simulink model "TosAeb"  (R2021a)
settings: Solver=FixedStepDiscrete  StartTime=0.0  StopTime=inf  FixedStep=0.01  RelTol=1e-3
undefined symbols (not defined in the file or in its folder): ACCModSts, AccSts1, AEB_ObjectMovingOrientation, AEB_ObjectMovingState, CN_BTCActive, CN_CurrentGearR, CN_CurrentGearR_DHT, CN_PTCActive, CN_VDCActive, CN_VSC_EspOff, CN_VSC_FCW_AEB_Error, CN_VSC_FCW_AEB_NoDispay, CN_VSC_FCW_AEB_PerfmDgdtn, CN_VSC_FCW_AEB_TempUnAvlbl, CN_VSC_VehStandstill, CNaccelAvoidMax, CNaccelAvoidMin, CNbrakePedalPress, CNcomfortStrLeftAccelMax, CNcomfortStrLeftAccelMin, CNcomfortStrRightAccelMax, CNcomfortStrRightAccelMin, CNDrvDoorOpen, CNDrvModeEco, CNDrvModeNorm, CNDrvModeOther, CNDrvModeSport, CNDrvSBRreminder, CNNull, CNobjInvalidID, CNPassDoorOpen, CNPassSBRreminder, CNPEBSAccelNegInf, CNPEBSAccelNull, CNPEBSTimeInf, CNPEBSTimeNull, CNSTOPMAX, CNSTOPNULL, CNsubjWidth, CNSwtOFF, CNSwtON, CNTTCMAX, CNTTCNULL, CNVehConfigAEBCycNone, CNVehConfigAEBNone, CNVehConfigAEBPedNone, CNVehConfigFCWNone, CNVehConfigJANone, CollisionSeverity, FALSE, FCWSnvty, JACycObjCalcInfoDefaultParams, JAPedObjCalcInfoDefaultParams, JAVehObjCalcInfoDefaultParams, latCycObjCalcInfoDefaultParams, latPedObjCalcInfoDefaultParams, longObjCalcInfoDefaultParams, ModSts, movingStatus, ObjectType ... (+1112)
libraries: simulink x3031, AEBSFunctionLib x50, Ta_Library x33, AEBSStateMachineLib x23, Mylibrary x12, simulink_extras x7, ObjSelect_lib x3, dspstat3 x2
(part 91 of 102)

== system TosAeb/AEB_Objsel/TA_Processor/AEB_ObjSelect/TargetInfoCheck/Data_precess/TA_EffectCalculate/Obj_FilterFlgCalculate/Long_ObjFilter/TTR_Calculate/CalculateTTRAndTTM_else/LongitudinalTTRComputer/CalculateTTI/StationaryHost/CheckIfSolutionExists/LimitedSafeDivide/Saturation Dynamic ==
  up : Inport
  u : Inport  Port=2
  lo : Inport  Port=3
  Data Type Duplicate : DataTypeDuplicate  DisableCoverage=on
  Data Type Propagation : S-Function  FunctionName=sfix_dtprop  Parameters=14,PropDataTypeMode,(getdatatypespecs(PropDataType,PropScaling,DblOver,0, 0, PropDataTypeMode, PropScalingMode)),IfRefDouble,IfRefSingle,IsSigned,NumBitsBase,NumBitsMult,NumBitsAdd,(sort(reshape(round(NumBitsAllowFinal), prod(size(NumBitsAllowFinal)),1))),PropScalingMode,ValuesUsedBestPrec,SlopeBase,SlopeMult,SlopeAdd,BiasBase,BiasMult,BiasAdd,DblOver  SFunctionDeploymentMode=off  EnableBusSupport=off  mask(Data Type Propagation){PropDataTypeMode=Inherit via propagation rule, PropDataType=fixdt(1, 16), IfRefDouble=double, IfRefSingle=single, IsSigned=IsSigned1, NumBitsBase=NumBits1, NumBitsMult=1, NumBitsAdd=0, NumBitsAllowFinal=1:128, PropScalingMode=Inherit via propagation rule, PropScaling=2^-10, ValuesUsedBestPrec=[5 -7], SlopeBase=Slope1, SlopeMult=1, SlopeAdd=0, BiasBase=Bias1, BiasMult=1, BiasAdd=0}
      mask prompts: PropDataTypeMode "Simulink:masks:x1PropagatedDataType_MP"; PropDataType "Simulink:masks:x11PropagatedDataTypeegFixdt116Fixdtsingle_MP"; IfRefDouble "Simulink:masks:x11IfAnyReferenceInputIsDoubleOutputIs_MP"; IfRefSingle "Simulink:masks:x12IfAnyReferenceInputIsSingleOutputIs_MP"; IsSigned "Simulink:masks:x13IsSigned_MP"; NumBitsBase "Simulink:masks:x141NumberofBitsBase_MP"; NumBitsMult "Simulink:masks:x142NumberofBitsMultiplicativeAdjustment_MP"; NumBitsAdd "Simulink:masks:x143NumberofBitsAdditiveAdjustment_MP"; NumBitsAllowFinal "Simulink:masks:x144NumberofBitsAllowableFinalValues_MP"; PropScalingMode "Simulink:masks:x2PropagatedScaling_MP"; PropScaling "Simulink:masks:x21PropagatedScalingSlopeEg29OrSlopeBiasEg1253_MP"; ValuesUsedBestPrec "Simulink:masks:x21ValuesUsedToDetermineBestPrecisionScaling_MP"; SlopeBase "Simulink:masks:x211SlopeBase_MP"; SlopeMult "Simulink:masks:x212SlopeMultiplicativeAdjustment_MP"; SlopeAdd "Simulink:masks:x213SlopeAdditiveAdjustment_MP"; BiasBase "Simulink:masks:x221BiasBase_MP"; BiasMult "Simulink:masks:x222BiasMultiplicativeAdjustment_MP"; BiasAdd "Simulink:masks:x223BiasAdditiveAdjustment_MP"
      mask help: Simulink:masks:DataTypePropagation_MD
      mask init:
      | DblOver = 0;
      mask TypeOptions PropDataTypeMode: Simulink:masks:SpecifyViaDialog | Simulink:masks:InheritViaPropagationRule
      mask Callback PropDataTypeMode: fixptdialog(gcbh)
      mask Callback PropDataType: fixptdialog(gcbh)
      mask TypeOptions IfRefDouble: Simulink:masks:double | Simulink:masks:single
      mask TypeOptions IfRefSingle: Simulink:masks:double | Simulink:masks:single
      mask TypeOptions IsSigned: Simulink:masks:IsSigned1 | Simulink:masks:IsSigned2 | Simulink:masks:IsSigned1OrIsSigned2 | Simulink:masks:TRUE | Simulink:masks:FALSE
      mask TypeOptions NumBitsBase: NumBits1 | NumBits2 | max([NumBits1 NumBits2]) | min([NumBits1 NumBits2]) | NumBits1+NumBits2
      mask TypeOptions PropScalingMode: Simulink:masks:SpecifyViaDialog | Simulink:masks:InheritViaPropagationRule | Simulink:masks:ObtainViaBestPrecision
      mask Callback PropScalingMode: fixptdialog(gcbh)
      mask TypeOptions SlopeBase: Slope1 | Slope2 | max([Slope1 Slope2]) | min([Slope1 Slope2]) | Slope1*Slope2 | Slope1/Slope2 | PosRange1 | PosRange2 | max([PosRange1 PosRange2]) | min([PosRange1 PosRange2]) | PosRange1*PosRange2 | PosRange1/PosRange2
      mask TypeOptions BiasBase: Bias1 | Bias2 | max([Bias1 Bias2]) | min([Bias1 Bias2]) | Bias1*Bias2 | Bias1/Bias2 | Bias1+Bias2 | Bias1-Bias2
      mask Display:
      | port_label('Input',1,'Ref1');
      | port_label('Input',2,'Ref2');
      | port_label('Input',3,'Prop');
  LowerRelop1 : RelationalOperator  DisableCoverage=on  Operator=>  OutDataTypeStr=boolean  RndMeth=Nearest
  Switch : Switch  Criteria=u2 ~= 0  OutDataTypeStr=Inherit: Inherit via back propagation  ZeroCross=off
  Switch2 : Switch  Criteria=u2 ~= 0  OutMin=OutMin  OutMax=OutMax  OutDataTypeStr=Inherit: Inherit via back propagation  ZeroCross=off
  UpperRelop : RelationalOperator  DisableCoverage=on  Operator=<  OutDataTypeStr=boolean  RndMeth=Nearest
  y : Outport  OutMin=OutMin  OutMax=OutMax
  wires:
  up/1 -> Switch2/1, LowerRelop1/2
  u/1 -> Data Type Propagation/2, Data Type Propagation/1, LowerRelop1/1, Switch/3, UpperRelop/1
  lo/1 -> Switch/1, UpperRelop/2
  Switch/1 -> Data Type Duplicate/2, Switch2/3, Data Type Propagation/3
  Switch2/1 -> Data Type Duplicate/1, y/1
  LowerRelop1/1 -> Switch2/2
  UpperRelop/1 -> Switch/2

== system TosAeb/AEB_Objsel/TA_Processor/AEB_ObjSelect/TargetInfoCheck/Data_precess/TA_EffectCalculate/Obj_FilterFlgCalculate/Long_ObjFilter/TTR_Calculate/CalculateTTRAndTTM_else/LongitudinalTTRComputer/CalculateTTI/StationaryHost/CheckIfSolutionExists1/LimitedSafeDivide/SafeDivide ==
  Numerator : Inport  OutDataTypeStr=DataType
      settings: AttributesFormatString=DT=%<OutDataTypeStr>
  Denominator : Inport  Port=2  OutDataTypeStr=DataType
      settings: AttributesFormatString=DT=%<OutDataTypeStr>
  SafeDivide : MATLAB Function
  Quotient : Outport  OutDataTypeStr=DataType
      settings: AttributesFormatString=DT=%<OutDataTypeStr>
  wires:
  SafeDivide/1(Quotient) -> Quotient/1
  Denominator/1 -> SafeDivide/2(Denominator)  "Denominator"
  Numerator/1 -> SafeDivide/1(Numerator)  "Numerator"

== system TosAeb/AEB_Objsel/TA_Processor/AEB_ObjSelect/TargetInfoCheck/Data_precess/TA_EffectCalculate/Obj_FilterFlgCalculate/Long_ObjFilter/TTR_Calculate/CalculateTTRAndTTM_else/LongitudinalTTRComputer/CalculateTTI/StationaryHost/CheckIfSolutionExists1/LimitedSafeDivide/Saturation Dynamic ==
  up : Inport
  u : Inport  Port=2
  lo : Inport  Port=3
  Data Type Duplicate : DataTypeDuplicate  DisableCoverage=on
  Data Type Propagation : S-Function  FunctionName=sfix_dtprop  Parameters=14,PropDataTypeMode,(getdatatypespecs(PropDataType,PropScaling,DblOver,0, 0, PropDataTypeMode, PropScalingMode)),IfRefDouble,IfRefSingle,IsSigned,NumBitsBase,NumBitsMult,NumBitsAdd,(sort(reshape(round(NumBitsAllowFinal), prod(size(NumBitsAllowFinal)),1))),PropScalingMode,ValuesUsedBestPrec,SlopeBase,SlopeMult,SlopeAdd,BiasBase,BiasMult,BiasAdd,DblOver  SFunctionDeploymentMode=off  EnableBusSupport=off  mask(Data Type Propagation){PropDataTypeMode=Inherit via propagation rule, PropDataType=fixdt(1, 16), IfRefDouble=double, IfRefSingle=single, IsSigned=IsSigned1, NumBitsBase=NumBits1, NumBitsMult=1, NumBitsAdd=0, NumBitsAllowFinal=1:128, PropScalingMode=Inherit via propagation rule, PropScaling=2^-10, ValuesUsedBestPrec=[5 -7], SlopeBase=Slope1, SlopeMult=1, SlopeAdd=0, BiasBase=Bias1, BiasMult=1, BiasAdd=0}
      mask prompts: PropDataTypeMode "Simulink:masks:x1PropagatedDataType_MP"; PropDataType "Simulink:masks:x11PropagatedDataTypeegFixdt116Fixdtsingle_MP"; IfRefDouble "Simulink:masks:x11IfAnyReferenceInputIsDoubleOutputIs_MP"; IfRefSingle "Simulink:masks:x12IfAnyReferenceInputIsSingleOutputIs_MP"; IsSigned "Simulink:masks:x13IsSigned_MP"; NumBitsBase "Simulink:masks:x141NumberofBitsBase_MP"; NumBitsMult "Simulink:masks:x142NumberofBitsMultiplicativeAdjustment_MP"; NumBitsAdd "Simulink:masks:x143NumberofBitsAdditiveAdjustment_MP"; NumBitsAllowFinal "Simulink:masks:x144NumberofBitsAllowableFinalValues_MP"; PropScalingMode "Simulink:masks:x2PropagatedScaling_MP"; PropScaling "Simulink:masks:x21PropagatedScalingSlopeEg29OrSlopeBiasEg1253_MP"; ValuesUsedBestPrec "Simulink:masks:x21ValuesUsedToDetermineBestPrecisionScaling_MP"; SlopeBase "Simulink:masks:x211SlopeBase_MP"; SlopeMult "Simulink:masks:x212SlopeMultiplicativeAdjustment_MP"; SlopeAdd "Simulink:masks:x213SlopeAdditiveAdjustment_MP"; BiasBase "Simulink:masks:x221BiasBase_MP"; BiasMult "Simulink:masks:x222BiasMultiplicativeAdjustment_MP"; BiasAdd "Simulink:masks:x223BiasAdditiveAdjustment_MP"
      mask help: Simulink:masks:DataTypePropagation_MD
      mask init:
      | DblOver = 0;
      mask TypeOptions PropDataTypeMode: Simulink:masks:SpecifyViaDialog | Simulink:masks:InheritViaPropagationRule
      mask Callback PropDataTypeMode: fixptdialog(gcbh)
      mask Callback PropDataType: fixptdialog(gcbh)
      mask TypeOptions IfRefDouble: Simulink:masks:double | Simulink:masks:single
      mask TypeOptions IfRefSingle: Simulink:masks:double | Simulink:masks:single
      mask TypeOptions IsSigned: Simulink:masks:IsSigned1 | Simulink:masks:IsSigned2 | Simulink:masks:IsSigned1OrIsSigned2 | Simulink:masks:TRUE | Simulink:masks:FALSE
      mask TypeOptions NumBitsBase: NumBits1 | NumBits2 | max([NumBits1 NumBits2]) | min([NumBits1 NumBits2]) | NumBits1+NumBits2
      mask TypeOptions PropScalingMode: Simulink:masks:SpecifyViaDialog | Simulink:masks:InheritViaPropagationRule | Simulink:masks:ObtainViaBestPrecision
      mask Callback PropScalingMode: fixptdialog(gcbh)
      mask TypeOptions SlopeBase: Slope1 | Slope2 | max([Slope1 Slope2]) | min([Slope1 Slope2]) | Slope1*Slope2 | Slope1/Slope2 | PosRange1 | PosRange2 | max([PosRange1 PosRange2]) | min([PosRange1 PosRange2]) | PosRange1*PosRange2 | PosRange1/PosRange2
      mask TypeOptions BiasBase: Bias1 | Bias2 | max([Bias1 Bias2]) | min([Bias1 Bias2]) | Bias1*Bias2 | Bias1/Bias2 | Bias1+Bias2 | Bias1-Bias2
      mask Display:
      | port_label('Input',1,'Ref1');
      | port_label('Input',2,'Ref2');
      | port_label('Input',3,'Prop');
  LowerRelop1 : RelationalOperator  DisableCoverage=on  Operator=>  OutDataTypeStr=boolean  RndMeth=Nearest
  Switch : Switch  Criteria=u2 ~= 0  OutDataTypeStr=Inherit: Inherit via back propagation  ZeroCross=off
  Switch2 : Switch  Criteria=u2 ~= 0  OutMin=OutMin  OutMax=OutMax  OutDataTypeStr=Inherit: Inherit via back propagation  ZeroCross=off
  UpperRelop : RelationalOperator  DisableCoverage=on  Operator=<  OutDataTypeStr=boolean  RndMeth=Nearest
  y : Outport  OutMin=OutMin  OutMax=OutMax
  wires:
  up/1 -> Switch2/1, LowerRelop1/2
  u/1 -> Data Type Propagation/2, Data Type Propagation/1, LowerRelop1/1, Switch/3, UpperRelop/1
  lo/1 -> Switch/1, UpperRelop/2
  Switch/1 -> Data Type Duplicate/2, Switch2/3, Data Type Propagation/3
  Switch2/1 -> Data Type Duplicate/1, y/1
  LowerRelop1/1 -> Switch2/2
  UpperRelop/1 -> Switch/2

== system TosAeb/AEB_Objsel/TA_Processor/AEB_ObjSelect/TargetInfoCheck/Data_precess/TA_EffectCalculate/Obj_FilterFlgCalculate/Long_ObjFilter/TTR_Calculate/CalculateTTRAndTTM_else/LongitudinalTTRComputer/CalculateTTI/StationaryHost/TimeToIntersectionComputer/SolutionChooser/Compare To Zero1 ==
  u : Inport
  Compare : RelationalOperator  Operator=<  OutDataTypeStr=boolean  RndMeth=Nearest  InputSameDT=on
  Constant : Constant  DisableCoverage=on  Value=0  OutDataTypeStr=Inherit: Inherit via back propagation  PreserveConstantTs=on
  y : Outport
  wires:
  Constant/1 -> Compare/2
  u/1 -> Compare/1
  Compare/1 -> y/1

== system TosAeb/AEB_Objsel/TA_Processor/AEB_ObjSelect/TargetInfoCheck/Data_precess/TA_EffectCalculate/Obj_FilterFlgCalculate/Long_ObjFilter/TTR_Calculate/CalculateTTRAndTTM_else/LongitudinalTTRComputer/CalculateTTI/StationaryHost/TimeToIntersectionComputer1/SolutionChooser/Compare To Zero1 ==
  u : Inport
  Compare : RelationalOperator  Operator=<  OutDataTypeStr=boolean  RndMeth=Nearest  InputSameDT=on
  Constant : Constant  DisableCoverage=on  Value=0  OutDataTypeStr=Inherit: Inherit via back propagation  PreserveConstantTs=on
  y : Outport
  wires:
  Compare/1 -> y/1
  u/1 -> Compare/1
  Constant/1 -> Compare/2

== system TosAeb/AEB_Objsel/TA_Processor/AEB_ObjSelect/TargetInfoCheck/Data_precess/TA_EffectCalculate/Obj_FilterFlgCalculate/Long_ObjFilter/TTR_Calculate/CalculateTTRAndTTM_else/LongitudinalTTRComputer/CalculateTTI/StationaryObject/CheckIfSolutionExists/LimitedSafeDivide/SafeDivide ==
  Numerator : Inport  OutDataTypeStr=DataType
      settings: AttributesFormatString=DT=%<OutDataTypeStr>
  Denominator : Inport  Port=2  OutDataTypeStr=DataType
      settings: AttributesFormatString=DT=%<OutDataTypeStr>
  SafeDivide : MATLAB Function
  Quotient : Outport  OutDataTypeStr=DataType
      settings: AttributesFormatString=DT=%<OutDataTypeStr>
  wires:
  SafeDivide/1(Quotient) -> Quotient/1
  Denominator/1 -> SafeDivide/2(Denominator)  "Denominator"
  Numerator/1 -> SafeDivide/1(Numerator)  "Numerator"

== system TosAeb/AEB_Objsel/TA_Processor/AEB_ObjSelect/TargetInfoCheck/Data_precess/TA_EffectCalculate/Obj_FilterFlgCalculate/Long_ObjFilter/TTR_Calculate/CalculateTTRAndTTM_else/LongitudinalTTRComputer/CalculateTTI/StationaryObject/CheckIfSolutionExists/LimitedSafeDivide/Saturation Dynamic ==
  up : Inport
  u : Inport  Port=2
  lo : Inport  Port=3
  Data Type Duplicate : DataTypeDuplicate  DisableCoverage=on
  Data Type Propagation : S-Function  FunctionName=sfix_dtprop  Parameters=14,PropDataTypeMode,(getdatatypespecs(PropDataType,PropScaling,DblOver,0, 0, PropDataTypeMode, PropScalingMode)),IfRefDouble,IfRefSingle,IsSigned,NumBitsBase,NumBitsMult,NumBitsAdd,(sort(reshape(round(NumBitsAllowFinal), prod(size(NumBitsAllowFinal)),1))),PropScalingMode,ValuesUsedBestPrec,SlopeBase,SlopeMult,SlopeAdd,BiasBase,BiasMult,BiasAdd,DblOver  SFunctionDeploymentMode=off  EnableBusSupport=off  mask(Data Type Propagation){PropDataTypeMode=Inherit via propagation rule, PropDataType=fixdt(1, 16), IfRefDouble=double, IfRefSingle=single, IsSigned=IsSigned1, NumBitsBase=NumBits1, NumBitsMult=1, NumBitsAdd=0, NumBitsAllowFinal=1:128, PropScalingMode=Inherit via propagation rule, PropScaling=2^-10, ValuesUsedBestPrec=[5 -7], SlopeBase=Slope1, SlopeMult=1, SlopeAdd=0, BiasBase=Bias1, BiasMult=1, BiasAdd=0}
      mask prompts: PropDataTypeMode "Simulink:masks:x1PropagatedDataType_MP"; PropDataType "Simulink:masks:x11PropagatedDataTypeegFixdt116Fixdtsingle_MP"; IfRefDouble "Simulink:masks:x11IfAnyReferenceInputIsDoubleOutputIs_MP"; IfRefSingle "Simulink:masks:x12IfAnyReferenceInputIsSingleOutputIs_MP"; IsSigned "Simulink:masks:x13IsSigned_MP"; NumBitsBase "Simulink:masks:x141NumberofBitsBase_MP"; NumBitsMult "Simulink:masks:x142NumberofBitsMultiplicativeAdjustment_MP"; NumBitsAdd "Simulink:masks:x143NumberofBitsAdditiveAdjustment_MP"; NumBitsAllowFinal "Simulink:masks:x144NumberofBitsAllowableFinalValues_MP"; PropScalingMode "Simulink:masks:x2PropagatedScaling_MP"; PropScaling "Simulink:masks:x21PropagatedScalingSlopeEg29OrSlopeBiasEg1253_MP"; ValuesUsedBestPrec "Simulink:masks:x21ValuesUsedToDetermineBestPrecisionScaling_MP"; SlopeBase "Simulink:masks:x211SlopeBase_MP"; SlopeMult "Simulink:masks:x212SlopeMultiplicativeAdjustment_MP"; SlopeAdd "Simulink:masks:x213SlopeAdditiveAdjustment_MP"; BiasBase "Simulink:masks:x221BiasBase_MP"; BiasMult "Simulink:masks:x222BiasMultiplicativeAdjustment_MP"; BiasAdd "Simulink:masks:x223BiasAdditiveAdjustment_MP"
      mask help: Simulink:masks:DataTypePropagation_MD
      mask init:
      | DblOver = 0;
      mask TypeOptions PropDataTypeMode: Simulink:masks:SpecifyViaDialog | Simulink:masks:InheritViaPropagationRule
      mask Callback PropDataTypeMode: fixptdialog(gcbh)
      mask Callback PropDataType: fixptdialog(gcbh)
      mask TypeOptions IfRefDouble: Simulink:masks:double | Simulink:masks:single
      mask TypeOptions IfRefSingle: Simulink:masks:double | Simulink:masks:single
      mask TypeOptions IsSigned: Simulink:masks:IsSigned1 | Simulink:masks:IsSigned2 | Simulink:masks:IsSigned1OrIsSigned2 | Simulink:masks:TRUE | Simulink:masks:FALSE
      mask TypeOptions NumBitsBase: NumBits1 | NumBits2 | max([NumBits1 NumBits2]) | min([NumBits1 NumBits2]) | NumBits1+NumBits2
      mask TypeOptions PropScalingMode: Simulink:masks:SpecifyViaDialog | Simulink:masks:InheritViaPropagationRule | Simulink:masks:ObtainViaBestPrecision
      mask Callback PropScalingMode: fixptdialog(gcbh)
      mask TypeOptions SlopeBase: Slope1 | Slope2 | max([Slope1 Slope2]) | min([Slope1 Slope2]) | Slope1*Slope2 | Slope1/Slope2 | PosRange1 | PosRange2 | max([PosRange1 PosRange2]) | min([PosRange1 PosRange2]) | PosRange1*PosRange2 | PosRange1/PosRange2
      mask TypeOptions BiasBase: Bias1 | Bias2 | max([Bias1 Bias2]) | min([Bias1 Bias2]) | Bias1*Bias2 | Bias1/Bias2 | Bias1+Bias2 | Bias1-Bias2
      mask Display:
      | port_label('Input',1,'Ref1');
      | port_label('Input',2,'Ref2');
      | port_label('Input',3,'Prop');
  LowerRelop1 : RelationalOperator  DisableCoverage=on  Operator=>  OutDataTypeStr=boolean  RndMeth=Nearest
  Switch : Switch  Criteria=u2 ~= 0  OutDataTypeStr=Inherit: Inherit via back propagation  ZeroCross=off
  Switch2 : Switch  Criteria=u2 ~= 0  OutMin=OutMin  OutMax=OutMax  OutDataTypeStr=Inherit: Inherit via back propagation  ZeroCross=off
  UpperRelop : RelationalOperator  DisableCoverage=on  Operator=<  OutDataTypeStr=boolean  RndMeth=Nearest
  y : Outport  OutMin=OutMin  OutMax=OutMax
  wires:
  up/1 -> Switch2/1, LowerRelop1/2
  u/1 -> Data Type Propagation/2, Data Type Propagation/1, LowerRelop1/1, Switch/3, UpperRelop/1
  lo/1 -> Switch/1, UpperRelop/2
  Switch/1 -> Data Type Duplicate/2, Switch2/3, Data Type Propagation/3
  Switch2/1 -> Data Type Duplicate/1, y/1
  LowerRelop1/1 -> Switch2/2
  UpperRelop/1 -> Switch/2

== system TosAeb/AEB_Objsel/TA_Processor/AEB_ObjSelect/TargetInfoCheck/Data_precess/TA_EffectCalculate/Obj_FilterFlgCalculate/Long_ObjFilter/TTR_Calculate/CalculateTTRAndTTM_else/LongitudinalTTRComputer/CalculateTTI/StationaryObject/CheckIfSolutionExists1/LimitedSafeDivide/SafeDivide ==
  Numerator : Inport  OutDataTypeStr=DataType
      settings: AttributesFormatString=DT=%<OutDataTypeStr>
  Denominator : Inport  Port=2  OutDataTypeStr=DataType
      settings: AttributesFormatString=DT=%<OutDataTypeStr>
  SafeDivide : MATLAB Function
  Quotient : Outport  OutDataTypeStr=DataType
      settings: AttributesFormatString=DT=%<OutDataTypeStr>
  wires:
  SafeDivide/1(Quotient) -> Quotient/1
  Denominator/1 -> SafeDivide/2(Denominator)  "Denominator"
  Numerator/1 -> SafeDivide/1(Numerator)  "Numerator"

== system TosAeb/AEB_Objsel/TA_Processor/AEB_ObjSelect/TargetInfoCheck/Data_precess/TA_EffectCalculate/Obj_FilterFlgCalculate/Long_ObjFilter/TTR_Calculate/CalculateTTRAndTTM_else/LongitudinalTTRComputer/CalculateTTI/StationaryObject/CheckIfSolutionExists1/LimitedSafeDivide/Saturation Dynamic ==
  up : Inport
  u : Inport  Port=2
  lo : Inport  Port=3
  Data Type Duplicate : DataTypeDuplicate  DisableCoverage=on
  Data Type Propagation : S-Function  FunctionName=sfix_dtprop  Parameters=14,PropDataTypeMode,(getdatatypespecs(PropDataType,PropScaling,DblOver,0, 0, PropDataTypeMode, PropScalingMode)),IfRefDouble,IfRefSingle,IsSigned,NumBitsBase,NumBitsMult,NumBitsAdd,(sort(reshape(round(NumBitsAllowFinal), prod(size(NumBitsAllowFinal)),1))),PropScalingMode,ValuesUsedBestPrec,SlopeBase,SlopeMult,SlopeAdd,BiasBase,BiasMult,BiasAdd,DblOver  SFunctionDeploymentMode=off  EnableBusSupport=off  mask(Data Type Propagation){PropDataTypeMode=Inherit via propagation rule, PropDataType=fixdt(1, 16), IfRefDouble=double, IfRefSingle=single, IsSigned=IsSigned1, NumBitsBase=NumBits1, NumBitsMult=1, NumBitsAdd=0, NumBitsAllowFinal=1:128, PropScalingMode=Inherit via propagation rule, PropScaling=2^-10, ValuesUsedBestPrec=[5 -7], SlopeBase=Slope1, SlopeMult=1, SlopeAdd=0, BiasBase=Bias1, BiasMult=1, BiasAdd=0}
      mask prompts: PropDataTypeMode "Simulink:masks:x1PropagatedDataType_MP"; PropDataType "Simulink:masks:x11PropagatedDataTypeegFixdt116Fixdtsingle_MP"; IfRefDouble "Simulink:masks:x11IfAnyReferenceInputIsDoubleOutputIs_MP"; IfRefSingle "Simulink:masks:x12IfAnyReferenceInputIsSingleOutputIs_MP"; IsSigned "Simulink:masks:x13IsSigned_MP"; NumBitsBase "Simulink:masks:x141NumberofBitsBase_MP"; NumBitsMult "Simulink:masks:x142NumberofBitsMultiplicativeAdjustment_MP"; NumBitsAdd "Simulink:masks:x143NumberofBitsAdditiveAdjustment_MP"; NumBitsAllowFinal "Simulink:masks:x144NumberofBitsAllowableFinalValues_MP"; PropScalingMode "Simulink:masks:x2PropagatedScaling_MP"; PropScaling "Simulink:masks:x21PropagatedScalingSlopeEg29OrSlopeBiasEg1253_MP"; ValuesUsedBestPrec "Simulink:masks:x21ValuesUsedToDetermineBestPrecisionScaling_MP"; SlopeBase "Simulink:masks:x211SlopeBase_MP"; SlopeMult "Simulink:masks:x212SlopeMultiplicativeAdjustment_MP"; SlopeAdd "Simulink:masks:x213SlopeAdditiveAdjustment_MP"; BiasBase "Simulink:masks:x221BiasBase_MP"; BiasMult "Simulink:masks:x222BiasMultiplicativeAdjustment_MP"; BiasAdd "Simulink:masks:x223BiasAdditiveAdjustment_MP"
      mask help: Simulink:masks:DataTypePropagation_MD
      mask init:
      | DblOver = 0;
      mask TypeOptions PropDataTypeMode: Simulink:masks:SpecifyViaDialog | Simulink:masks:InheritViaPropagationRule
      mask Callback PropDataTypeMode: fixptdialog(gcbh)
      mask Callback PropDataType: fixptdialog(gcbh)
      mask TypeOptions IfRefDouble: Simulink:masks:double | Simulink:masks:single
      mask TypeOptions IfRefSingle: Simulink:masks:double | Simulink:masks:single
      mask TypeOptions IsSigned: Simulink:masks:IsSigned1 | Simulink:masks:IsSigned2 | Simulink:masks:IsSigned1OrIsSigned2 | Simulink:masks:TRUE | Simulink:masks:FALSE
      mask TypeOptions NumBitsBase: NumBits1 | NumBits2 | max([NumBits1 NumBits2]) | min([NumBits1 NumBits2]) | NumBits1+NumBits2
      mask TypeOptions PropScalingMode: Simulink:masks:SpecifyViaDialog | Simulink:masks:InheritViaPropagationRule | Simulink:masks:ObtainViaBestPrecision
      mask Callback PropScalingMode: fixptdialog(gcbh)
      mask TypeOptions SlopeBase: Slope1 | Slope2 | max([Slope1 Slope2]) | min([Slope1 Slope2]) | Slope1*Slope2 | Slope1/Slope2 | PosRange1 | PosRange2 | max([PosRange1 PosRange2]) | min([PosRange1 PosRange2]) | PosRange1*PosRange2 | PosRange1/PosRange2
      mask TypeOptions BiasBase: Bias1 | Bias2 | max([Bias1 Bias2]) | min([Bias1 Bias2]) | Bias1*Bias2 | Bias1/Bias2 | Bias1+Bias2 | Bias1-Bias2
      mask Display:
      | port_label('Input',1,'Ref1');
      | port_label('Input',2,'Ref2');
      | port_label('Input',3,'Prop');
  LowerRelop1 : RelationalOperator  DisableCoverage=on  Operator=>  OutDataTypeStr=boolean  RndMeth=Nearest
  Switch : Switch  Criteria=u2 ~= 0  OutDataTypeStr=Inherit: Inherit via back propagation  ZeroCross=off
  Switch2 : Switch  Criteria=u2 ~= 0  OutMin=OutMin  OutMax=OutMax  OutDataTypeStr=Inherit: Inherit via back propagation  ZeroCross=off
  UpperRelop : RelationalOperator  DisableCoverage=on  Operator=<  OutDataTypeStr=boolean  RndMeth=Nearest
  y : Outport  OutMin=OutMin  OutMax=OutMax
  wires:
  up/1 -> Switch2/1, LowerRelop1/2
  u/1 -> Data Type Propagation/2, Data Type Propagation/1, LowerRelop1/1, Switch/3, UpperRelop/1
  lo/1 -> Switch/1, UpperRelop/2
  Switch/1 -> Data Type Duplicate/2, Switch2/3, Data Type Propagation/3
  Switch2/1 -> Data Type Duplicate/1, y/1
  LowerRelop1/1 -> Switch2/2
  UpperRelop/1 -> Switch/2

== system TosAeb/AEB_Objsel/TA_Processor/AEB_ObjSelect/TargetInfoCheck/Data_precess/TA_EffectCalculate/Obj_FilterFlgCalculate/Long_ObjFilter/TTR_Calculate/CalculateTTRAndTTM_else/LongitudinalTTRComputer/CalculateTTI/StationaryObject/TimeToIntersectionComputer/SolutionChooser/Compare To Zero1 ==
  u : Inport
  Compare : RelationalOperator  Operator=<  OutDataTypeStr=boolean  RndMeth=Nearest  InputSameDT=on
  Constant : Constant  DisableCoverage=on  Value=0  OutDataTypeStr=Inherit: Inherit via back propagation  PreserveConstantTs=on
  y : Outport
  wires:
  Constant/1 -> Compare/2
  u/1 -> Compare/1
  Compare/1 -> y/1

== system TosAeb/AEB_Objsel/TA_Processor/AEB_ObjSelect/TargetInfoCheck/Data_precess/TA_EffectCalculate/Obj_FilterFlgCalculate/Long_ObjFilter/TTR_Calculate/CalculateTTRAndTTM_else/LongitudinalTTRComputer/CalculateTTI/StationaryObject/TimeToIntersectionComputer1/SolutionChooser/Compare To Zero1 ==
  u : Inport
  Compare : RelationalOperator  Operator=<  OutDataTypeStr=boolean  RndMeth=Nearest  InputSameDT=on
  Constant : Constant  DisableCoverage=on  Value=0  OutDataTypeStr=Inherit: Inherit via back propagation  PreserveConstantTs=on
  y : Outport
  wires:
  Compare/1 -> y/1
  u/1 -> Compare/1
  Constant/1 -> Compare/2

== system TosAeb/AEB_Objsel/TA_Processor/AEB_ObjSelect/TargetInfoCheck/Data_precess/TA_EffectCalculate/ValideateObj/ObjPos_Calculate/ObjPos_Calculateelse/InPath_Cal/DetermineInPath/PredictHostLateralPositionAtTTR/CalculateHeading/ExtractVelocitiesAtStartOfSegments/ExtractVelocityVectorFirstPoint/SegmentComponents ==
  Segment : Inport  OutDataTypeStr=Bus:PahEstimn
  Bus Selector : BusSelector  OutputSignals=CtrlAryLgt,CtrlAryLat
  Selector : Selector  InputPortWidth=4  IndexOptions=Index vector (dialog)  Indices=1  OutputSizes=1
  Selector1 : Selector  InputPortWidth=4  IndexOptions=Index vector (dialog)  Indices=2  OutputSizes=1
  Selector2 : Selector  InputPortWidth=4  IndexOptions=Index vector (dialog)  Indices=3  OutputSizes=1
  Selector3 : Selector  InputPortWidth=4  IndexOptions=Index vector (dialog)  Indices=4  OutputSizes=1
  Selector4 : Selector  InputPortWidth=4  IndexOptions=Index vector (dialog)  Indices=1  OutputSizes=1
  Selector5 : Selector  InputPortWidth=4  IndexOptions=Index vector (dialog)  Indices=2  OutputSizes=1
  Selector6 : Selector  InputPortWidth=4  IndexOptions=Index vector (dialog)  Indices=3  OutputSizes=1
  Selector7 : Selector  InputPortWidth=4  IndexOptions=Index vector (dialog)  Indices=4  OutputSizes=1
  P0x : Outport
  P1x : Outport  Port=2
  P2x : Outport  Port=3
  P3x : Outport  Port=4
  P0y : Outport  Port=5
  P1y : Outport  Port=6
  P2y : Outport  Port=7
  P3y : Outport  Port=8
  wires:
  Bus Selector/2 -> Selector4/1, Selector5/1, Selector6/1, Selector7/1  "<CtrlAryLat>"
  Selector7/1 -> P3y/1  "P3y"
  Selector6/1 -> P2y/1  "P2y"
  Selector5/1 -> P1y/1  "P1y"
  Selector4/1 -> P0y/1  "P0y"
  Selector3/1 -> P3x/1  "P3x"
  Selector2/1 -> P2x/1  "P2x"
  Selector1/1 -> P1x/1  "P1x"
  Selector/1 -> P0x/1  "P0x"
  Bus Selector/1 -> Selector2/1, Selector1/1, Selector/1, Selector3/1  "<CtrlAryLgt>"
  Segment/1 -> Bus Selector/1

== stateflow chart TosAeb/FcwAebJa_Step_sys/ALG_AEBSystem/ALG_AEBController/AEB_LatObjInfoCalcApp/LatPedObjCalc/Subsystem/latPedObjInfoCalc/ObjColiisionRiskProbCalc/CollisionRiskProbCalc/ObjReginCorrCalc/ObjLengthWidthCalc/ObjLengthWidthCalc ==
  (default) -> (junction 10) : {
      | 	ObjLengthCorr = P_LatPedCA_CollisionRiskProbLengthThres;
      | 	ObjWidthCorr = P_LatPedCA_CollisionRiskProbWidthThres;
      | }
  (junction 10) -> (junction 12) [order 1] : [(abs(ObjectYawAngle) > 45 &&...
      | abs(ObjectYawAngle) < 135)||...
      | ObjectMovingOrientation == AEB_ObjectMovingOrientation.Crossing]
  (junction 10) -> (junction 16) [order 2]
  (junction 12) -> (junction 14) : {
      | 	ObjLengthCorr = ObjectWidth;
      | 	ObjWidthCorr = ObjectLength;
      | }
  (junction 14) -> (junction 16)
  (junction 16) -> (junction 19)
  data: ObjectWidth input; ObjectYawAngle input; ObjectMovingOrientation input; ObjectLength input; ObjLengthCorr output single; ObjWidthCorr output single; P_LatPedCA_CollisionRiskProbLengthThres input; P_LatPedCA_CollisionRiskProbWidthThres input

== stateflow chart TosAeb/FcwAebJa_Step_sys/ALG_AEBSystem/ALG_AEBController/AEB_LatObjInfoCalcApp/LatPedObjCalc/Subsystem/latPedObjInfoCalc/ObjColiisionRiskProbCalc/CollisionRiskProbCalc/ObjReginCorrCalc/ObjLengthWidthCalc/ObjLengthWidthCalc1 ==
  (default) -> (junction 32)
  (junction 32) -> (junction 34) [order 1] : [ObjectTypeCameraConfirmed == true]
  (junction 32) -> (junction 30) [order 2] : {
      | 	ObjectLength = P_LatPedCA_CollisionRiskProbLengthThres;
      | 	ObjectWidth = P_LatPedCA_CollisionRiskProbWidthThres;
      | }
  (junction 34) -> (junction 36) : {
      | 	ObjectLength = ObjectLengthIn;
      | 	ObjectWidth = ObjectWidthIn;
      | }
  (junction 36) -> (junction 30)
  (junction 30) -> (junction 10)
  (junction 10) -> (junction 12) [order 1] : [(abs(ObjectYawAngle) > 45 &&...
      | abs(ObjectYawAngle) < 135)||...
      | ObjectMovingOrientation == AEB_ObjectMovingOrientation.Crossing]
  (junction 10) -> (junction 16) [order 2]
  (junction 12) -> (junction 14) : {
      |       temp = ObjectLength;
      | 	ObjectLength = ObjectWidth;
      | 	ObjectWidth = temp;
      | }
  (junction 14) -> (junction 16)
  (junction 16) -> (junction 19) : {
      |    ObjectLength = min(ObjectLength,1.5);
      |    ObjectWidth = min(ObjectWidth,1.5);
      | }
  data: ObjectTypeCameraConfirmed input boolean; ObjectLengthIn input; ObjectWidthIn input; ObjectYawAngle input; ObjectMovingOrientation input; ObjectLength output single; ObjectWidth output single; P_LatPedCA_CollisionRiskProbLengthThres input; P_LatPedCA_CollisionRiskProbWidthThres input; temp local single

== stateflow chart TosAeb/FcwAebJa_Step_sys/ALG_AEBSystem/ALG_AEBInput/LongObjInfoMap/MovingStatus_Subsystem/MovingStatusSwitch ==
  (default) -> (junction 83)
  (junction 83) -> (junction 85) [order 1] : [Input_Moving==1]
  (junction 83) -> (junction 91) [order 2]
  (junction 85) -> (junction 87) : {MovingStatus=3;}
  (junction 91) -> (junction 93) [order 1] : [Input_Stopped==1]
  (junction 91) -> (junction 100) [order 2]
  (junction 93) -> (junction 95) : {MovingStatus=2;}
  (junction 100) -> (junction 102) [order 1] : [Input_Stationary==1]
  (junction 100) -> (junction 21) [order 2] : {MovingStatus=0;}
  (junction 102) -> (junction 104) : {MovingStatus=1;}
  (junction 104) -> (junction 21)
  (junction 95) -> (junction 104)
  (junction 87) -> (junction 95)
  (junction 21) -> (junction 25)
  data: Input_Unknown input; Input_Stationary input; Input_Stopped input; MovingStatus output uint8; Input_Moving input

== stateflow chart TosAeb/FcwAebJa_Step_sys/ALG_AEBSystem/ALG_AEBInput/LongObjInfoMap/Subsystem/MovingStatus_Subsystem/MovingStatusSwitch ==
  (default) -> (junction 83)
  (junction 83) -> (junction 85) [order 1] : [Input_Moving==1]
  (junction 83) -> (junction 91) [order 2]
  (junction 85) -> (junction 87) : {MovingStatus=3;}
  (junction 91) -> (junction 93) [order 1] : [Input_Stopped==1]
  (junction 91) -> (junction 100) [order 2]
  (junction 93) -> (junction 95) : {MovingStatus=2;}
  (junction 100) -> (junction 102) [order 1] : [Input_Stationary==1]
  (junction 100) -> (junction 21) [order 2] : {MovingStatus=0;}
  (junction 102) -> (junction 104) : {MovingStatus=1;}
  (junction 104) -> (junction 21)
  (junction 95) -> (junction 104)
  (junction 87) -> (junction 95)
  (junction 21) -> (junction 25)
  data: Input_Unknown input; Input_Stationary input; Input_Stopped input; MovingStatus output uint8; Input_Moving input

== stateflow chart TosAeb/FcwAebJa_Step_sys/ALG_AEBSystem/ALG_AEBInput/LongObjInfoMap/Subsystem1/MovingStatus_Subsystem/MovingStatusSwitch ==
  (default) -> (junction 83)
  (junction 83) -> (junction 85) [order 1] : [Input_Moving==1]
  (junction 83) -> (junction 91) [order 2]
  (junction 85) -> (junction 87) : {MovingStatus=3;}
  (junction 91) -> (junction 93) [order 1] : [Input_Stopped==1]
  (junction 91) -> (junction 100) [order 2]
  (junction 93) -> (junction 95) : {MovingStatus=2;}
  (junction 100) -> (junction 102) [order 1] : [Input_Stationary==1]
  (junction 100) -> (junction 21) [order 2] : {MovingStatus=0;}
  (junction 102) -> (junction 104) : {MovingStatus=1;}
  (junction 104) -> (junction 21)
  (junction 95) -> (junction 104)
  (junction 87) -> (junction 95)
  (junction 21) -> (junction 25)
  data: Input_Unknown input; Input_Stationary input; Input_Stopped input; MovingStatus output uint8; Input_Moving input

== stateflow chart TosAeb/FcwAebJa_Step_sys/ALG_AEBSystem/ALG_AEBController/AEB_FuncImpManagementApp/FuncManeuArbCalc/brkFuncManeuArbCalc/axTgtReqValueCalc1/axTgtReqValueCalc4AebControl/JerkFuncDecCalc/JerkFuncDecCalc/JerkFuncDecCalc ==
  state JerkFuncDec = JerkFuncDecCalc(aDesired,axTgtReqValueK1,accelMin,JerkFuncDecK1,JerkFuncDecMax) [function]
  state JerkFuncDecFirstCycle = JerkFuncDecFirstCycleCalc(aDesired,JerkFuncDecMax) [function]
  (default) -> (junction 77)
  (junction 77) -> (junction 81) [order 1] : [JerkFuncDecReset == true]
  (junction 77) -> (junction 79) [order 2]
  (junction 81) -> (junction 83) : {
      |    JerkFuncDec = 0;
      | }
  (junction 83) -> (junction 79)
  (junction 79) -> (junction 6)
  (junction 6) -> (junction 8) [order 1] : [AebActiveFirstCycle == false]
  (junction 6) -> (junction 28) [order 2] : {
      |    JerkFuncDec = ...
      |    JerkFuncDecFirstCycleCalc(aDesired,JerkFuncDecMax);
      |    %shallFirstGradientUsed = false;
      | }
  (junction 8) -> (junction 10)
  (junction 10) -> (junction 12) [order 1] : [JerkDecHoldTime == false]
  (junction 10) -> (junction 16) [order 2] : {
      |   temp_JerkFuncDec = ...
      |   JerkFuncDecCalc(aDesired,axTgtReqValueK1,accelMin,JerkFuncDec,JerkFuncDecMax);
      | }
  (junction 12) -> (junction 14)
  (junction 16) -> (junction 18) [order 1] : [temp_JerkFuncDec  <  JerkFuncDec]
      | %&&...(freezeGradient == false)]
  (junction 16) -> (junction 22) [order 2]
  (junction 18) -> (junction 20) : {
      |   JerkFuncDec = temp_JerkFuncDec;
      | }
  (junction 20) -> (junction 22)
  (junction 22) -> (junction 25)
  (junction 25) -> (junction 28)
  (junction 14) -> (junction 25)
  (junction 28) -> (junction 31)
  data: aDesired function_input; axTgtReqValueK1 function_input; accelMin function_input; JerkFuncDecK1 function_input; JerkFuncDecMax function_input; JerkFuncDec function_output; aDesired function_input; JerkFuncDecMax function_input; JerkFuncDecFirstCycle function_output; JerkFuncDecReset input; JerkFuncDecMax input; aDesired input; accelMin input; axTgtReqValueK1 input; JerkDecHoldTime input; JerkFuncDec output single =0; temp_JerkFuncDec local single; AebActiveFirstCycle input

== stateflow chart TosAeb/FcwAebJa_Step_sys/ALG_AEBSystem/ALG_AEBController/AEB_LatObjInfoCalcApp/LatPedObjCalc/Subsystem/latPedObjInfoCalc/ObjColiisionRiskProbCalc/CollisionRiskProbCalc/ObjReginCorrCalc/PedMaxAccelCalc/PedMovingMode ==
  (default) -> (junction 2)
  (junction 2) -> (junction 3) [order 1] : [vxAbs < P_LatPedCA_CollisionRiskProbPedStopStsVelThres]
  (junction 2) -> (junction 7) [order 2]
  (junction 3) -> (junction 5) : {
      | 	pedMovingState = 0;
      | }
  (junction 7) -> (junction 9) [order 1] : [vxAbs < P_LatPedCA_CollisionRiskProbWalkingStsVelThres]
  (junction 7) -> (junction 14) [order 2]
  (junction 9) -> (junction 11) : {
      | 	pedMovingState = 1;
      | }
  (junction 14) -> (junction 16) [order 1] : [vxAbs < P_LatPedCA_CollisionRiskProbJoggingStsVelThres]
  (junction 14) -> (junction 20) [order 2] : {
      | 	pedMovingState = 3;
      | }
  (junction 16) -> (junction 18) : {
      | 	pedMovingState = 2;
      | }
  (junction 18) -> (junction 20)
  (junction 11) -> (junction 18)
  (junction 5) -> (junction 11)
  (junction 20) -> (junction 24)
  data: vxAbs input; P_LatPedCA_CollisionRiskProbPedStopStsVelThres input; pedMovingState output single; P_LatPedCA_CollisionRiskProbWalkingStsVelThres input; P_LatPedCA_CollisionRiskProbJoggingStsVelThres input

== stateflow chart TosAeb/FcwAebJa_Step_sys/ALG_AEBSystem/ALG_AEBController/AEB_JaObjCalcApp/JAVehTgtInfoCalc/AEBJAVehObjCalc/JAVehObjInfoCalc/SensorTgtInfo2AbsTgtInfoTrans/ObjFusQualityCal ==
  state [deltaDxThres,deltaDyThres] = SensorDxThres(subjVel) [function]
  (default) -> (junction 9)
  (junction 9) -> (junction 11) [order 1] : [ObjectID ~= CNobjInvalidID &&...
      | ObjectTypeCameraConfirmed == true]
  (junction 9) -> (junction 27) [order 2] : {
      |    ObjFusQuality = false;
      | }
  (junction 11) -> (junction 13)
  (junction 13) -> (junction 15) [order 1] : [FR_ObjectID ~= CNobjInvalidID &&...
      | FC_ObjectID ~= CNobjInvalidID]
  (junction 13) -> (junction 24) [order 2] : {
      |    ObjFusQuality = false;
      | }
  (junction 15) -> (junction 17) : {
      |         temp_Dx = abs(FR_Dx - FC_Dx);
      |         temp_Dy = abs(FR_Dy - FC_Dy);
      |         [deltaDxThres,deltaDyThres] = SensorDxThres(subjVel);
      | }
  (junction 17) -> (junction 19) [order 1] : [temp_Dx < deltaDxThres &&...
      | temp_Dy < deltaDyThres]
  (junction 17) -> (junction 33) [order 2] : {
      |    ObjFusQuality = false;
      | }
  (junction 19) -> (junction 21) : {
      |    ObjFusQuality = true;
      | }
  (junction 24) -> (junction 27)
  (junction 33) -> (junction 24)
  (junction 21) -> (junction 33)
  (junction 27) -> (junction 30)
  data: subjVel function_input; deltaDxThres function_output single; deltaDyThres function_output single; ObjectID input size=1; ObjectTypeCameraConfirmed input size=1; FC_ObjectID input size=1; FR_ObjectID input size=1; FR_Dy input size=1; FR_Dx input size=1; FC_Dx input size=1; FC_Dy input size=1; CNobjInvalidID parameter size=1; ObjFusQuality output size=1; temp_Dx local single size=1; temp_Dy local single size=1; deltaDyThres local single size=1 =single(256); deltaDxThres local single size=1 =single(256); subjVel input size=1

== stateflow chart TosAeb/FcwAebJa_Step_sys/ALG_AEBSystem/ALG_AEBController/AEB_JaObjCalcApp/JAPedTgtInfoCalc/JAPedObjCalcInfoCalc/JAPedObjInfoCalc/SensorTgtInfo2AbsTgtInfoTrans/ObjFusQualityCal ==
  state deltaDxThres = SensorDxThres(subjVel) [function]
  (default) -> (junction 9)
  (junction 9) -> (junction 11) [order 1] : [ObjectID ~= CNobjInvalidID &&...
      | ObjectTypeCameraConfirmed == true]
  (junction 9) -> (junction 27) [order 2] : {
      |    ObjFusQuality = false;
      | }
  (junction 11) -> (junction 13)
  (junction 13) -> (junction 15) [order 1] : [FR_ObjectID ~= CNobjInvalidID &&...
      | FC_ObjectID ~= CNobjInvalidID]
  (junction 13) -> (junction 24) [order 2] : {
      |    ObjFusQuality = false;
      | }
  (junction 15) -> (junction 17) : {
      |         temp_Dx = abs(FR_Dx - FC_Dx);
      |         deltaDxThres = SensorDxThres(subjVel);
      | }
  (junction 17) -> (junction 19) [order 1] : [temp_Dx < deltaDxThres]
  (junction 17) -> (junction 33) [order 2] : {
      |    ObjFusQuality = false;
      | }
  (junction 19) -> (junction 21) : {
      |    ObjFusQuality = true;
      | }
  (junction 24) -> (junction 27)
  (junction 33) -> (junction 24)
  (junction 21) -> (junction 33)
  (junction 27) -> (junction 30)
  data: subjVel function_input; deltaDxThres function_output single; ObjectID input size=1; ObjectTypeCameraConfirmed input size=1; FC_ObjectID input size=1; FR_ObjectID input size=1; FR_Dx input size=1; FC_Dx input size=1; CNobjInvalidID parameter size=1; ObjFusQuality output size=1; temp_Dx local single size=1; deltaDxThres local single size=1 =single(256); subjVel input size=1

== stateflow chart TosAeb/FcwAebJa_Step_sys/ALG_AEBSystem/ALG_AEBController/AEB_LatObjInfoCalcApp/LatPedObjCalc/LatPedObjCalc/latPedObjInfoCalc/SensorTgtInfo2AbsTgtInfoTrans/ObjFusQualityCal ==
  state [deltaDxThres,deltaDyThres,deltaVyThres] = SensorVyThres(subjVel) [function]
  (default) -> (junction 9)
  (junction 9) -> (junction 11) [order 1] : [ObjectID ~= CNobjInvalidID &&...
      | ObjectTypeCameraConfirmed == true]
  (junction 9) -> (junction 27) [order 2] : {
      |    ObjFusQuality = false;
      | }
  (junction 11) -> (junction 13)
  (junction 13) -> (junction 15) [order 1] : [FR_ObjectID ~= CNobjInvalidID &&...
      | FC_ObjectID ~= CNobjInvalidID]
  (junction 13) -> (junction 24) [order 2] : {
      |    ObjFusQuality = false;
      | }
  (junction 15) -> (junction 17) : {
      |         temp_Dx = abs(FR_Dx - FC_Dx);
      |         temp_Dy = abs(FR_Dy - FC_Dy);
      |         temp_Vy = abs(FR_Vy - FC_Vy);
      |         [deltaDxThres,deltaDyThres,deltaVyThres] = SensorVyThres(subjVel);
      | }
  (junction 17) -> (junction 19) [order 1] : [(abs(FR_Vy) < P_AEB_LatPedObjAbs_SensorFR_VyThres&&...
      | temp_Vy > deltaVyThres &&...
      | abs(dy) > P_AEB_LatPedObjAbs_DyThres4deltaVy)||...
      | temp_Dx > deltaDxThres||...
      | temp_Dy > deltaDyThres ]
  (junction 17) -> (junction 33) [order 2] : {
      |    ObjFusQuality = true;
      | }
  (junction 19) -> (junction 21) : {
      |    ObjFusQuality = false;
      | }
  (junction 24) -> (junction 27)
  (junction 33) -> (junction 24)
  (junction 21) -> (junction 33)
  (junction 27) -> (junction 30)
  data: deltaDxThres function_output single; deltaDyThres function_output single; subjVel function_input; deltaVyThres function_output single; ObjectID input size=1; ObjectTypeCameraConfirmed input size=1; dy input; FR_ObjectID input size=1; FR_Dx input; FR_Dy input; FR_Vy input; FC_ObjectID input size=1; FC_Dx input; FC_Dy input; CNobjInvalidID parameter size=1; ObjFusQuality output size=1; deltaVyThres local single size=1 =single(0); FC_Vy input; P_AEB_LatPedObjAbs_DyThres4deltaVy input; temp_Vy local single; P_AEB_LatPedObjAbs_SensorFR_VyThres input; subjVel input size=1; deltaDxThres local single =single(256); temp_Dx local single; deltaDyThres local single =single(256); temp_Dy local single

== stateflow chart TosAeb/FcwAebJa_Step_sys/ALG_AEBSystem/ALG_AEBController/AEB_LatObjInfoCalcApp/LatCycObjCalc/LatCycObjCalc/latCycObjInfoCalc/SensorTgtInfo2AbsTgtInfoTrans/ObjFusQualityCal ==
  state [deltaDxThres,deltaDyThres,deltaVyThres] = SensorVyThres(subjVel) [function]
  (default) -> (junction 9)
  (junction 9) -> (junction 11) [order 1] : [ObjectID ~= CNobjInvalidID &&...
      | ObjectTypeCameraConfirmed == true]
  (junction 9) -> (junction 27) [order 2] : {
      |    ObjFusQuality = false;
      | }
  (junction 11) -> (junction 13)
  (junction 13) -> (junction 15) [order 1] : [FR_ObjectID ~= CNobjInvalidID &&...
      | FC_ObjectID ~= CNobjInvalidID]
  (junction 13) -> (junction 24) [order 2] : {
      |    ObjFusQuality = false;
      | }
  (junction 15) -> (junction 17) : {
      |         temp_Dx = abs(FR_Dx - FC_Dx);
      |         temp_Dy = abs(FR_Dy - FC_Dy);
      |         temp_Vy = abs(FR_Vy - FC_Vy);
      |         [deltaDxThres,deltaDyThres,deltaVyThres] = SensorVyThres(subjVel);
      | }
  (junction 17) -> (junction 19) [order 1] : [(abs(FR_Vy) < P_AEB_LatCycObjAbs_SensorFR_VyThres&&...
      | temp_Vy > deltaVyThres &&...
      | abs(dy)   > P_AEB_LatCycObjAbs_DyThres4deltaVy)||...
      | temp_Dx > deltaDxThres||...
      | temp_Dy > deltaDyThres]
  (junction 17) -> (junction 33) [order 2] : {
      |    ObjFusQuality = true;
      | }
  (junction 19) -> (junction 21) : {
      |    ObjFusQuality = false;
      | }
  (junction 24) -> (junction 27)
  (junction 33) -> (junction 24)
  (junction 21) -> (junction 33)
  (junction 27) -> (junction 30)
  data: deltaDxThres function_output single; deltaDyThres function_output single; subjVel function_input; deltaVyThres function_output single; ObjectID input size=1; ObjectTypeCameraConfirmed input size=1; dy input; FR_ObjectID input size=1; FR_Dx input; FR_Dy input; FR_Vy input; FC_ObjectID input size=1; FC_Dx input; FC_Dy input; CNobjInvalidID parameter size=1; ObjFusQuality output size=1; deltaVyThres local single size=1 =single(0); FC_Vy input; P_AEB_LatCycObjAbs_DyThres4deltaVy input; temp_Vy local single; P_AEB_LatCycObjAbs_SensorFR_VyThres input; subjVel input size=1; deltaDxThres local single =single(256); temp_Dx local single; deltaDyThres local single =single(256); temp_Dy local single

== stateflow chart TosAeb/FcwAebJa_Step_sys/ALG_AEBSystem/ALG_AEBController/AEB_LatObjInfoCalcApp/LatPedObjCalc/Subsystem/latPedObjInfoCalc/ObjColiisionRiskProbCalc/CollisionRiskProbCalcFlow ==
  (default) -> (junction 11)
  (junction 11) -> (junction 2) : {
      | 	tPredictTime = 0;
      | 	temp_collisionRiskProbMax = 0;
      | }
  (junction 2) -> (junction 6) [order 1] : [tPredictTime < tMaxPredTime]
  (junction 41) -> (junction 2)
  (junction 2) -> (junction 13) [order 2] : {
      | 	collisionRiskProbMax = temp_collisionRiskProbMax;
      | 	preCollisonMaxTime = temp_preCollisonMaxTime;
      | 	dxCollisionRiskProbOut = dxCollisionRiskProb;
      | 	dyCollisionRiskProbOut = dyCollisionRiskProb;
      | }
  (junction 6) -> (junction 32) : {
      | 	tPredictTimeOut = tPredictTime;
      | 	CollisionRiskProbCalcFunc;
      | }
  (junction 32) -> (junction 34) [order 1] : [collisionRiskProb > temp_collisionRiskProbMax]
  (junction 32) -> (junction 8) [order 2]
  (junction 34) -> (junction 35) : {
      | 	temp_collisionRiskProbMax = collisionRiskProb;
      | 	temp_preCollisonMaxTime =  tPredictTime;
      | }
  (junction 35) -> (junction 8)
  (junction 8) -> (junction 41) : {
      | 	tPredictTime += stepTime;
      | }
  data: collisionRiskProbMax output single; dxCollisionRiskProbOut output single; dyCollisionRiskProbOut output single; preCollisonMaxTime output single; stepTime input single; tMaxPredTime input single; collisionRiskProb input single; dyCollisionRiskProb input single; tPredictTimeOut output single; tPredictTime local single; temp_collisionRiskProbMax local single; temp_preCollisonMaxTime local single; dxCollisionRiskProb input single
  events: CollisionRiskProbCalcFunc output FUNCTION_CALL_EVENT

== stateflow chart TosAeb/FcwAebJa_Step_sys/ALG_AEBSystem/ALG_AEBController/AEB_JaObjCalcApp/JaCycTgtInfoCalc/JaCycObjCalcInfoCalc/JaCycObjInfoCalc/ObjCrossFlgCal/CollisionProbCalc/Subsystem/ObjLenthWidthCalc/ObjLengthWidthCalc ==
  (default) -> (junction 2)
  (junction 2) -> (junction 3) [order 1] : [FusionType == true]
  (junction 2) -> (junction 7) [order 2] : {
      | 	ObjLengthCorr = 0.5;
      | 	ObjWidthCorr = 0.5;
      | }
  (junction 3) -> (junction 5) : {
      | 	ObjLengthCorr = ObjectLength;
      | 	ObjWidthCorr = ObjectWidth;
      | }
  (junction 5) -> (junction 7)
  (junction 7) -> (junction 10)
  (junction 10) -> (junction 12) [order 1] : [(abs(ObjectYawAngle) > 45 &&...
      | abs(ObjectYawAngle) < 135) ||...
      | objectMovingOrientation == AEB_ObjectMovingOrientation.Crossing]
  (junction 10) -> (junction 16) [order 2]
  (junction 12) -> (junction 14) : {
      | 	ObjLengthCorr = ObjectWidth;
      | 	ObjWidthCorr = ObjectLength;
      | }
  (junction 14) -> (junction 16)
  (junction 16) -> (junction 19)
  data: ObjectYawAngle input single; objectMovingOrientation input; ObjectLength input single; FusionType input; ObjectWidth input single; ObjLengthCorr output single; ObjWidthCorr output single

== stateflow chart TosAeb/FcwAebJa_Step_sys/ALG_AEBSystem/ALG_AEBController/AEB_JaObjCalcApp/JaCycTgtInfoCalc/JaCycObjCalcInfoCalc/JaCycObjInfoCalc/ObjCrossFlgCal/CollisionProbCalc/Subsystem/PreCrossFlgCalcFlow ==
  state preCrossFlg = VectorCrossAlgorithm(x1,y1,x2,y2,m1,n1,m2,n2) [function]
  (default) -> (junction 59) : {
      | 	preCrossFlg = false;
      | 	i = 0;
      | }
  (junction 59) -> (junction 58) [order 1] : [ i < 4]
  (junction 58) -> (junction 48) : {
      | 	p = (i+1)%%4;
      | 	j = 0;
      | }
  (junction 48) -> (junction 46) [order 1] : [j < 4]
  (junction 46) -> (junction 49) : {
      | 	q = (j+1)%%4;
      | 	preCrossFlg = VectorCrossAlgorithm(subjDxPre[i],subjDyPre[i],subjDxPre[p],subjDyPre[p],...
      | 	objDxPre[j],objDyPre[j],objDxPre[q],objDyPre[q]);
      | }
  (junction 60) -> (junction 59) : {
      | 	i++;
      | }
  (junction 59) -> (junction 61) [order 2]
  (junction 49) -> (junction 48) [order 2] : {
      | 	j++;
      | }
  (junction 48) -> (junction 60) [order 2]
  (junction 49) -> (junction 64) [order 1] : [preCrossFlg == true]
  (junction 64) -> (junction 61)
  (junction 61) -> (junction 67)
  data: x1 function_input single; y1 function_input single; x2 function_input single; y2 function_input single; m1 function_input single; n1 function_input single; m2 function_input single; n2 function_input single; preCrossFlg function_output; subjDxPre input single; subjDyPre input single; objDxPre input single; objDyPre input single; j local single; i local single; p local single; q local single; preCrossFlg output boolean

== stateflow chart TosAeb/FcwAebJa_Step_sys/ALG_AEBSystem/ALG_AEBController/AEB_JaObjCalcApp/JaCycTgtInfoCalc/JaCycObjCalcInfoCalc/JaCycObjInfoCalc/ObjCrossFlgCal/CollisionProbCalcFlow ==
  (default) -> (junction 11)
  (junction 11) -> (junction 2) : {tPredictTime = 0;}
  (junction 2) -> (junction 6) [order 1] : [tPredictTime <= P_JaPedCA_PreCollisionPredTimeMax]
  (junction 6) -> (junction 8) : {
      | 	tPredictTimeOut = tPredictTime;
      | 	CollisionProbFunc;
      | }
  (junction 8) -> (junction 2) [order 2] : {
      | 	tPredictTime += P_JaPedCA_PreCollisionStepTime;
      | }
  (junction 2) -> (junction 13) [order 2] : {
      |   TTC = single(256);
      | }
  (junction 8) -> (junction 22) [order 1] : [preCollisionProb == true]
  (junction 22) -> (junction 13) : {
      |   TTC = tPredictTimeOut;
      | }
  (junction 13) -> (junction 25)
  data: TTC output single; P_JaPedCA_PreCollisionStepTime input single; P_JaPedCA_PreCollisionPredTimeMax input single; tPredictTimeOut output single; tPredictTime local single; preCollisionProb input
  events: CollisionProbFunc output FUNCTION_CALL_EVENT

== stateflow chart TosAeb/FcwAebJa_Step_sys/ALG_AEBSystem/ALG_AEBController/AEB_LatObjInfoCalcApp/LatPedObjCalc/Subsystem/latPedObjInfoCalc/ObjCrossFlgCal/CollisionProbCalcFlow ==
  (default) -> (junction 11)
  (junction 11) -> (junction 2) : {
      |   tPredictTime = 0;
      |   TTC = 256;
      | }
  (junction 2) -> (junction 6) [order 1] : [tPredictTime < tMaxPredTime]
  (junction 30) -> (junction 2) [order 2]
  (junction 2) -> (junction 13) [order 2]
  (junction 6) -> (junction 8) : {
      | 	tPredictTimeOut = tPredictTime;
      | 	CollisionProbFunc;
      | }
  (junction 8) -> (junction 30) : {
      | 	tPredictTime += stepTime;
      | }
  (junction 30) -> (junction 22) [order 1] : [preCollisionProb == true]
  (junction 22) -> (junction 13) : {
      |   TTC = tPredictTimeOut;
      | }
  (junction 13) -> (junction 25)
  data: stepTime input single; tMaxPredTime input single; tPredictTimeOut output single; tPredictTime local single; preCollisionProb input; TTC output single
  events: CollisionProbFunc output FUNCTION_CALL_EVENT
